simulink model "HvacRecircCtrl"  (R2022b)
settings: Solver=FixedStepDiscrete  StartTime=0.0  StopTime=10.0  FixedStep=0.2  RelTol=1e-3
settings Model: Creator=blue.ge  LastModifiedBy=blue.ge
settings DataIOCC: SaveFormat=StructureWithTime
settings OptimizationCC: DefaultParameterBehavior=Inlined  EnhancedBackFolding=on  PassReuseOutputArgsAs=Individual arguments  ZeroExternalMemoryAtStartup=off  ZeroInternalMemoryAtStartup=off  OptimizeBlockOrder=speed  DifferentSizesBufferReuse=on  OptimizationCustomize=off  EfficientTunableParamExpr=on
settings DebuggingCC: ParameterTunabilityLossMsg=error
settings ModelReferenceCC: ModelReferenceNumInstancesAllowed=Single
settings SFSimCC: MATLABDynamicMemAlloc=off
settings RTWCC: Description=AUTOSAR  SystemTargetFile=autosar.tlc  GenCodeOnly=on  TemplateMakefile=ert_default_tmf
settings CodeAppCC: ForceParamTrailComments=on  IgnoreCustomStorageClasses=off  OperatorAnnotations=on  SimulinkDataObjDesc=on  SFDataObjDesc=on  InsertBlockDesc=on
settings STFCustomTargetCC: TargetLangStandard=C99 (ISO)  MultiwordLength=256  ParMdlRefBuildCompliant=on  CompOptLevelCompliant=on  IncludeMdlTerminateFcn=off  CombineOutputUpdateFcns=on  SuppressErrorStatus=on  ERTCustomFileBanners=on  SupportNonFinite=off  SupportComplex=off  SupportContinuousTime=off  SupportNonInlinedSFcns=off  RemoveResetFunc=on  MATLABClassNameForMDSCustomization=Simulink.SoftwareTarget.ERTCustomization  AutosarCompliant=on  UseToolchainInfoCompliant=on  LookupTableObjectStructAxisOrder=2,1,3,4,...  LUTObjectStructOrderEvenSpacing=Size,Table,Breakpoints  SystemTargetFile=autosar.tlc
settings ERTTargetCC: TargetLangStandard=C99 (ISO)  ConcurrentExecutionCompliant=off  IncludeMdlTerminateFcn=off  SuppressErrorStatus=on  SupportNonFinite=off  SupportComplex=off  ModelStepFunctionPrototypeControlCompliant=off  CPPClassGenCompliant=off  AutosarCompliant=on  LookupTableObjectStructAxisOrder=2,1,3,4,...  LUTObjectStructOrderEvenSpacing=Size,Table,Breakpoints  InlinedParameterPlacement=NonHierarchical  ERTCustomFileTemplate=example_file_process.tlc

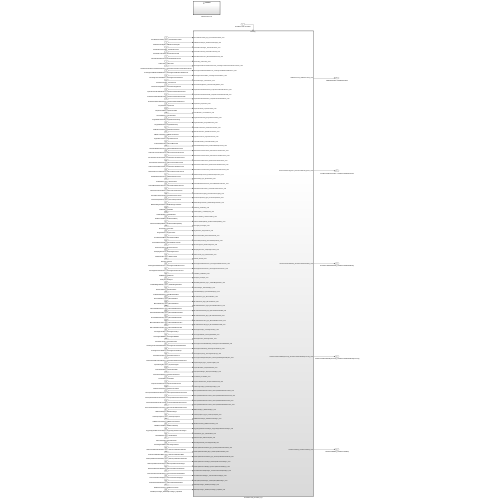
[thumbnail: Simulink's stored preview of the top-level diagram]
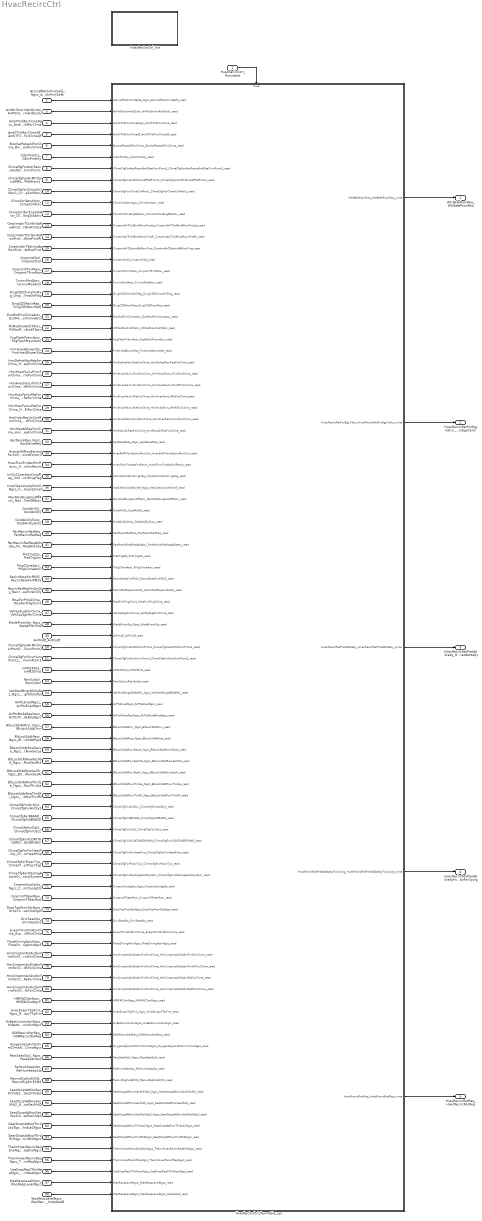
== system HvacRecircCtrl ==
  HvacRecircCtrl_Runnable : Inport  SampleTime=0.2  OutputFunctionCall=on
  ActvnOfWshrFrntSafe_Ngcc_ActvnOfWshrFrntSafe : Inport  Port=2  OutDataTypeStr=Enum: OnOffCrit1  PortDimensions=1
  AirMtrlSnsrInAirQlyIdx_AirMtrlSnsrInAirQlyIdx : Inport  Port=3  OutDataTypeStr=AirMtrlSnsrAirQlyIdx  PortDimensions=1
  AmbTFildForClimaNgcc_AmbTFildForClima : Inport  Port=4  OutDataTypeStr=T30  PortDimensions=1
  AmbTFildForClimaQf_AmbTFildForClimaQf : Inport  Port=5  OutDataTypeStr=Enum: GenQf1  PortDimensions=1
  BiochalPatseldForClima_BiochalPatseldForClima : Inport  Port=6  OutDataTypeStr=Boolean  PortDimensions=1
  CdsnProblty_CdsnProblty : Inport  Port=7  OutDataTypeStr=Fac30  PortDimensions=1
  ClimaCfgForActrRecircAndOsaCmnPrsnt1_ClimaCfgForActrRecircAndOsaCmnPrsnt1 : Inport  Port=8  OutDataTypeStr=Enum: ClimaCfgForActrRecircAndOsaCmnPrsnt1  PortDimensions=1
  ClimaCfgForAirMtrlSnsrAPMAPrsnt1_ClimaCfgForAirMtrlSnsrAPMAPrsnt1 : Inport  Port=9  OutDataTypeStr=Boolean  PortDimensions=1
  ClimaCfgForClimaCtrlMan1_ClimaCfgForClimaCtrlMan1 : Inport  Port=10  OutDataTypeStr=Boolean  PortDimensions=1
  ClimaCtrlActvNgcc_ClimaCtrlActv : Inport  Port=11  OutDataTypeStr=Boolean  PortDimensions=1
  ClimaCtrlForEngStbActv_ClimaCtrlForEngStbActv : Inport  Port=12  OutDataTypeStr=Boolean  PortDimensions=1
  CmptmtAirTCtrlErrAtRowFirstLe_CmptmtAirTCtrlErrAtRowFirstLe : Inport  Port=13  OutDataTypeStr=T30  PortDimensions=1
  CmptmtAirTCtrlErrAtRowFirstRi_CmptmtAirTCtrlErrAtRowFirstRi : Inport  Port=14  OutDataTypeStr=T30  PortDimensions=1
  CmptmtAirTEstimdAtRowFirst_CmptmtAirTEstimdAtRowFirst : Inport  Port=15  OutDataTypeStr=T30  PortDimensions=1
  CmptmtClsd_CmptmtClsd : Inport  Port=16  OutDataTypeStr=Boolean  PortDimensions=1
  CmptmtTFrntRaw_CmptmtTFrntRaw : Inport  Port=17  OutDataTypeStr=Bus: CmptmtTFrnt  PortDimensions=1  BusOutputAsStruct=on
  CnvincModActv_CnvincModActv : Inport  Port=18  OutDataTypeStr=Boolean  PortDimensions=1
  DrvgCDSClimaOnFlag_DrvgCDSClimaOnFlag : Inport  Port=19  OutDataTypeStr=Boolean  PortDimensions=1
  DrvgCDSRecirReq_DrvgCDSRecirReq : Inport  Port=20  OutDataTypeStr=Perc30  PortDimensions=1
  EcoModForClimaActv_EcoModForClimaActv : Inport  Port=21  OutDataTypeStr=Enum: OnOff1  PortDimensions=1
  FltModForAmbTActv_FltModForAmbTActv : Inport  Port=22  OutDataTypeStr=Boolean  PortDimensions=1
  FogFlashPrevnActv_FogFlashPrevnActv : Inport  Port=23  OutDataTypeStr=Boolean  PortDimensions=1
  FrntHvacBlowerSts_FrntHvacBlowerSts : Inport  Port=24  OutDataTypeStr=Enum: HvacFanSts  PortDimensions=1
  HmiDefrstMaxReqForClima_HmiDefrstMaxReqForClima : Inport  Port=25  OutDataTypeStr=Enum: ActrReq  PortDimensions=1
  HmiHvacFanLvlFrntForClima_HmiHvacFanLvlFrntForClima : Inport  Port=26  OutDataTypeStr=Enum: HmiHvacFanLvl  PortDimensions=1
  HmiHvacFanLvlFrntForClima_HmiHvacFanLvlFrntRiForClima : Inport  Port=27  OutDataTypeStr=Enum: HmiHvacFanLvl  PortDimensions=1
  HmiHvacFanLvlReForClima_HmiHvacFanLvlReForClima : Inport  Port=28  OutDataTypeStr=Enum: HmiHvacFanLvl  PortDimensions=1
  HmiHvacFanLvlReForClima_HmiHvacFanLvlReRiForClima : Inport  Port=29  OutDataTypeStr=Enum: HmiHvacFanLvl  PortDimensions=1
  HmiHvacRecircCmdForClima_HmiHvacRecircCmdForClima : Inport  Port=30  OutDataTypeStr=Enum: HmiHvacRecircCmd  PortDimensions=1
  HmiMaxACReqForClima_HmiMaxACReqForClima : Inport  Port=31  OutDataTypeStr=Enum: OnOff1  PortDimensions=1
  HpcDeiceReq_Ngcc_HpcDeiceReq : Inport  Port=32  OutDataTypeStr=Enum: DeiceReq  PortDimensions=1
  HvacAirMFlowEstimdForCtrlr_HvacAirMFlowEstimdForCtrlr : Inport  Port=33  OutDataTypeStr=AirFlow30  PortDimensions=1
  HvacFlowFrntActForRecirc_HvacFlowFrntActForRecirc : Inport  Port=34  OutDataTypeStr=AirFlow30  PortDimensions=1
  IntCo2SnsrActvDrvgFlag_IntCo2SnsrActvDrvgFlag : Inport  Port=35  OutDataTypeStr=Boolean  PortDimensions=1
  InteCleanUnpleSmell_Ngcc_InteCleanUnpleSmell : Inport  Port=36  OutDataTypeStr=Enum: OnOff1  PortDimensions=1
  ManModDurgFanOffActv_ManModDurgFanOffActv : Inport  Port=37  OutDataTypeStr=Boolean  PortDimensions=1
  OutdAirQly_OutdAirQly : Inport  Port=38  OutDataTypeStr=Bus: OutdAirQly  PortDimensions=1  BusOutputAsStruct=on
  OutdAirQlyDuty_OutdAirQlyDuty : Inport  Port=39  OutDataTypeStr=Bus: OutdAirQlyDutyWithQly  PortDimensions=1  BusOutputAsStruct=on
  PartRecircRatReq_PartRecircRatReq : Inport  Port=40  OutDataTypeStr=Perc30  PortDimensions=1
  PartRecircRatReqAtStaby_PartRecircRatReqAtStaby : Inport  Port=41  OutDataTypeStr=Perc30  PortDimensions=1
  PreClngSts_PreClngSts : Inport  Port=42  OutDataTypeStr=Enum: PreClngSts  PortDimensions=1
  PrkgClimaActv_PrkgClimaActv : Inport  Port=43  OutDataTypeStr=Enum: PrkgClimaActv  PortDimensions=1
  RecircRateForPM25_RecircRateForPM25 : Inport  Port=44  OutDataTypeStr=Perc30  PortDimensions=1
  RecircRatReqForAirQly_RecircRatReqForAirQly : Inport  Port=45  OutDataTypeStr=Perc30  PortDimensions=1
  RtLeForPrkgClima_RtLeForPrkgClima : Inport  Port=46  OutDataTypeStr=Ti33  PortDimensions=1
  VehSpdLgtForClima_VehSpdLgtForClima : Inport  Port=47  OutDataTypeStr=Bus: VehSpdLgtForClima  PortDimensions=1  BusOutputAsStruct=on
  WadeProtnSts_Ngcc_WadeProtnSts : Inport  Port=48  OutDataTypeStr=Enum: OnOff1  PortDimensions=1
  AirPmQf_AirPmQf : Inport  Port=49  OutDataTypeStr=Enum: AirPmQf  PortDimensions=1
  ClimaCfgForAirMtrlSnsrPrsnt1_ClimaCfgForAirMtrlSnsrPrsnt1 : Inport  Port=50  OutDataTypeStr=Enum: CarCfgForAirMtrlSnsrGly  PortDimensions=1
  ClimaCfgForSnsrHumPrsnt1_ClimaCfgForSnsrHumPrsnt1 : Inport  Port=51  OutDataTypeStr=Boolean  PortDimensions=1
  InPM25Fild_InPM25Fild : Inport  Port=52  OutDataTypeStr=UInt10  PortDimensions=1
  RainSybol_RainSybol : Inport  Port=53  OutDataTypeStr=Boolean  PortDimensions=1
  VehModMngtGlbSafe1_Ngcc_VehModMngtGlbSafe1 : Inport  Port=54  OutDataTypeStr=Bus: VehModMngtGlbSafe1  PortDimensions=1  BusOutputAsStruct=on
  AirPtcEnadNgcc_AirPtcEnadNgcc : Inport  Port=55  OutDataTypeStr=Boolean  PortDimensions=1
  AirPtcModeReqNgcc_AirPtcModeReqNgcc : Inport  Port=56  OutDataTypeStr=Enum: AirPtcModeReq  PortDimensions=1
  BltLockStAtDrvr_Ngcc_BltLockStAtDrvr : Inport  Port=57  OutDataTypeStr=Bus: BltLockStFrnt  PortDimensions=1  BusOutputAsStruct=on
  BltLockStAtPass_Ngcc_BltLockStAtPass : Inport  Port=58  OutDataTypeStr=Bus: BltLockStFrnt  PortDimensions=1  BusOutputAsStruct=on
  BltLockStAtRowSecLe_Ngcc_BltLockStAtRowSecLe : Inport  Port=59  OutDataTypeStr=Bus: BltLockStRe  PortDimensions=1  BusOutputAsStruct=on
  BltLockStAtRowSecMid_Ngcc_BltLockStAtRowSecMid : Inport  Port=60  OutDataTypeStr=Bus: BltLockStRe  PortDimensions=1  BusOutputAsStruct=on
  BltLockStAtRowSecRi_Ngcc_BltLockStAtRowSecRi : Inport  Port=61  OutDataTypeStr=Bus: BltLockStRe  PortDimensions=1  BusOutputAsStruct=on
  BltLockStAtRowThrdLe_Ngcc_BltLockStAtRowThrdLe : Inport  Port=62  OutDataTypeStr=Bus: BltLockStRe  PortDimensions=1  BusOutputAsStruct=on
  BltLockStAtRowThrdRi_Ngcc_BltLockStAtRowThrdRi : Inport  Port=63  OutDataTypeStr=Bus: BltLockStRe  PortDimensions=1  BusOutputAsStruct=on
  ClimaCfgForAirQly1_ClimaCfgForAirQly1 : Inport  Port=64  OutDataTypeStr=Boolean  PortDimensions=1
  ClimaCfgForBRAND_ClimaCfgForBRAND : Inport  Port=65  OutDataTypeStr=Enum: ClimaCfgForBRAND  PortDimensions=1
  ClimaCfgForCds1_ClimaCfgForCds1 : Inport  Port=66  OutDataTypeStr=Boolean  PortDimensions=1
  ClimaCfgForFLOATINGDRVING_ClimaCfgForFLOATINGDRVING : Inport  Port=67  OutDataTypeStr=Enum: FLOATINGDRVING  PortDimensions=1
  ClimaCfgForForHeatPmp_ClimaCfgForForHeatPmp : Inport  Port=68  OutDataTypeStr=Enum: CarCfgForHeatPmpTyp  PortDimensions=1
  ClimaCfgForPrpsnTyp_ClimaCfgForPrpsnTyp : Inport  Port=69  OutDataTypeStr=Enum: CarCfgForPrpsnTypGly  PortDimensions=1
  ClimaCfgForWadingAssistSystem_ClimaCfgForWadingAssistSystem : Inport  Port=70  OutDataTypeStr=Enum: WadingAssistSystem  PortDimensions=1
  CmptmtCoolgSts_Ngcc_CmptmtCoolgSts : Inport  Port=71  OutDataTypeStr=Enum: CmptmtCoolgSts  PortDimensions=1
  CmptmtTRearRaw_CmptmtTRearRaw : Inport  Port=72  OutDataTypeStr=Bus: CmptmtTFrnt  PortDimensions=1  BusOutputAsStruct=on
  DropTopPosnStsNgcc_DropTopPosnStsNgcc : Inport  Port=73  OutDataTypeStr=Enum: PosnSts  PortDimensions=1
  DrvrSeatSts_DrvrSeatSts : Inport  Port=74  OutDataTypeStr=Enum: OccptPresSt1  PortDimensions=1
  EvaprTFrntFildForClima_EvaprTFrntFildForClima : Inport  Port=75  OutDataTypeStr=T30  PortDimensions=1
  FloatDrvingActvNgcc_FloatDrvingActvNgcc : Inport  Port=76  OutDataTypeStr=Bus: FloatDrvingActv  PortDimensions=1  BusOutputAsStruct=on
  HmiCmptmtAirDistbnFrntForClima_HmiCmptmtAirDistbnFrntForClima : Inport  Port=77  OutDataTypeStr=Enum: HmiCmptmtAirDistbnFrnt  PortDimensions=1
  HmiCmptmtAirDistbnFrntForClima_HmiCmptmtAirDistbnFrntRiForClima : Inport  Port=78  OutDataTypeStr=Enum: HmiCmptmtAirDistbnFrnt  PortDimensions=1
  HmiCmptmtAirDistbnFrntForClima_HmiCmptmtAirDistbnReForClima : Inport  Port=79  OutDataTypeStr=Enum: HmiCmptmtAirDistbnFrnt  PortDimensions=1
  HmiCmptmtAirDistbnFrntForClima_HmiCmptmtAirDistbnReRiForClima : Inport  Port=80  OutDataTypeStr=Enum: HmiCmptmtAirDistbnFrnt  PortDimensions=1
  HMIFACSwtNgcc_HMIFACSwtNgcc : Inport  Port=81  OutDataTypeStr=Enum: OnOff1  PortDimensions=1
  HvacEvaprTSpFrnt_Ngcc_HvacEvaprTSpFrnt : Inport  Port=82  OutDataTypeStr=HvacEvaprTSp  PortDimensions=1
  HvBattLimnIndcnNgcc_HvBattLimnIndcnNgcc : Inport  Port=83  OutDataTypeStr=UInt16  PortDimensions=1
  OSMRecircRatReq_OSMRecircRatReq : Inport  Port=84  OutMin=0  OutMax=2047  OutDataTypeStr=Pwr30  PortDimensions=1
  OxygenSplyAvlStsFrmClimaNgcc_OxygenSplyAvlStsFrmClimaNgcc : Inport  Port=85  OutDataTypeStr=Enum: OxygenSplyAvlSts  PortDimensions=1
  PassSeatSts2_Ngcc_PassSeatSts2 : Inport  Port=86  OutDataTypeStr=Enum: OccptPresSt1  PortDimensions=1
  RelHumKeepSts_RelHumKeepSts : Inport  Port=87  OutDataTypeStr=Enum: RelHumKeepSts  PortDimensions=1
  ResrvdSigForECM4_ResrvdSigForECM4 : Inport  Port=88  OutDataTypeStr=BattSnsrChksTbl  PortDimensions=1
  SeatOccptAtRowSecDrSide2_Ngcc_SeatOccptAtRowSecDrSide2 : Inport  Port=89  OutDataTypeStr=Enum: OccptPresSt1  PortDimensions=1
  SeatOccptAtRowSecMid2_Ngcc_SeatOccptAtRowSecMid2 : Inport  Port=90  OutDataTypeStr=Enum: OccptPresSt1  PortDimensions=1
  SeatOccptAtRowSecPasSide2_Ngcc_SeatOccptAtRowSecPasSide2 : Inport  Port=91  OutDataTypeStr=Enum: OccptPresSt1  PortDimensions=1
  SeatOccptAtRowThrdLe2Ngcc_SeatOccptAtRowThrdLe2Ngcc : Inport  Port=92  OutDataTypeStr=Enum: OccptPresSt1  PortDimensions=1
  SeatOccptAtRowThrdRi2Ngcc_SeatOccptAtRowThrdRi2Ngcc : Inport  Port=93  OutDataTypeStr=Enum: OccptPresSt1  PortDimensions=1
  ThermHvacRecircReqEnaNgcc_ThermHvacRecircReqEnaNgcc : Inport  Port=94  OutDataTypeStr=Boolean  PortDimensions=1
  ThermHvacRecircReqNgcc_ThermHvacRecircReqNgcc : Inport  Port=95  OutMin=0  OutMax=100  OutDataTypeStr=Perc6  PortDimensions=1
  UseEvapReqTMinNewNgcc_UseEvapReqTMinNewNgcc : Inport  Port=96  OutDataTypeStr=Enum: OnOff1  PortDimensions=1
  WasRespLevelNgcc_WasRespLevelNgcc : Inport  Port=97  OutDataTypeStr=Enum: WasRespLevel  PortDimensions=1
  WasRespLevelNgcc_WasRespLevelNgcc_IsUpdated : Inport  Port=98  OutDataTypeStr=boolean  PortDimensions=1
  HvacRecircCtrl_Init : Subsystem
  HvacRecircCtrl_Runnable_sys : Subsystem
  WtrBafePsonReq_WtrBafePsonReq : Outport  OutDataTypeStr=UInt16  PortDimensions=1
  HvacRecircRatForEgyCalcn_HvacRecircRatForEgyCalcn : Outport  Port=2  OutDataTypeStr=Perc30  PortDimensions=1
  HvacRecircRatPredAtStaby_HvacRecircRatPredAtStaby : Outport  Port=3  OutDataTypeStr=Perc30  PortDimensions=1
  HvacRecircRatPredAtStabyForCoolg_HvacRecircRatPredAtStabyForCoolg : Outport  Port=4  OutDataTypeStr=Perc30  PortDimensions=1
  HvacRecircRatReq_HvacRecircRatReq : Outport  Port=5  OutDataTypeStr=Perc30  PortDimensions=1
  wires:
  ActvnOfWshrFrntSafe_Ngcc_ActvnOfWshrFrntSafe/1 -> HvacRecircCtrl_Runnable_sys/1(ActvnOfWshrFrntSafe_Ngcc_ActvnOfWshrFrntSafe_read)
  AirMtrlSnsrInAirQlyIdx_AirMtrlSnsrInAirQlyIdx/1 -> HvacRecircCtrl_Runnable_sys/2(AirMtrlSnsrInAirQlyIdx_AirMtrlSnsrInAirQlyIdx_read)
  AmbTFildForClimaNgcc_AmbTFildForClima/1 -> HvacRecircCtrl_Runnable_sys/3(AmbTFildForClimaNgcc_AmbTFildForClima_read)
  AmbTFildForClimaQf_AmbTFildForClimaQf/1 -> HvacRecircCtrl_Runnable_sys/4(AmbTFildForClimaQf_AmbTFildForClimaQf_read)
  BiochalPatseldForClima_BiochalPatseldForClima/1 -> HvacRecircCtrl_Runnable_sys/5(BiochalPatseldForClima_BiochalPatseldForClima_read)
  CdsnProblty_CdsnProblty/1 -> HvacRecircCtrl_Runnable_sys/6(CdsnProblty_CdsnProblty_read)
  ClimaCfgForActrRecircAndOsaCmnPrsnt1_ClimaCfgForActrRecircAndOsaCmnPrsnt1/1 -> HvacRecircCtrl_Runnable_sys/7(ClimaCfgForActrRecircAndOsaCmnPrsnt1_ClimaCfgForActrRecircAndOsaCmnPrsnt1_read)
  ClimaCfgForAirMtrlSnsrAPMAPrsnt1_ClimaCfgForAirMtrlSnsrAPMAPrsnt1/1 -> HvacRecircCtrl_Runnable_sys/8(ClimaCfgForAirMtrlSnsrAPMAPrsnt1_ClimaCfgForAirMtrlSnsrAPMAPrsnt1_read)
  ClimaCfgForClimaCtrlMan1_ClimaCfgForClimaCtrlMan1/1 -> HvacRecircCtrl_Runnable_sys/9(ClimaCfgForClimaCtrlMan1_ClimaCfgForClimaCtrlMan1_read)
  ClimaCtrlActvNgcc_ClimaCtrlActv/1 -> HvacRecircCtrl_Runnable_sys/10(ClimaCtrlActvNgcc_ClimaCtrlActv_read)
  ClimaCtrlForEngStbActv_ClimaCtrlForEngStbActv/1 -> HvacRecircCtrl_Runnable_sys/11(ClimaCtrlForEngStbActv_ClimaCtrlForEngStbActv_read)
  CmptmtAirTCtrlErrAtRowFirstLe_CmptmtAirTCtrlErrAtRowFirstLe/1 -> HvacRecircCtrl_Runnable_sys/12(CmptmtAirTCtrlErrAtRowFirstLe_CmptmtAirTCtrlErrAtRowFirstLe_read)
  CmptmtAirTCtrlErrAtRowFirstRi_CmptmtAirTCtrlErrAtRowFirstRi/1 -> HvacRecircCtrl_Runnable_sys/13(CmptmtAirTCtrlErrAtRowFirstRi_CmptmtAirTCtrlErrAtRowFirstRi_read)
  CmptmtAirTEstimdAtRowFirst_CmptmtAirTEstimdAtRowFirst/1 -> HvacRecircCtrl_Runnable_sys/14(CmptmtAirTEstimdAtRowFirst_CmptmtAirTEstimdAtRowFirst_read)
  CmptmtClsd_CmptmtClsd/1 -> HvacRecircCtrl_Runnable_sys/15(CmptmtClsd_CmptmtClsd_read)
  CmptmtTFrntRaw_CmptmtTFrntRaw/1 -> HvacRecircCtrl_Runnable_sys/16(CmptmtTFrntRaw_CmptmtTFrntRaw_read)
  CnvincModActv_CnvincModActv/1 -> HvacRecircCtrl_Runnable_sys/17(CnvincModActv_CnvincModActv_read)
  DrvgCDSClimaOnFlag_DrvgCDSClimaOnFlag/1 -> HvacRecircCtrl_Runnable_sys/18(DrvgCDSClimaOnFlag_DrvgCDSClimaOnFlag_read)
  DrvgCDSRecirReq_DrvgCDSRecirReq/1 -> HvacRecircCtrl_Runnable_sys/19(DrvgCDSRecirReq_DrvgCDSRecirReq_read)
  EcoModForClimaActv_EcoModForClimaActv/1 -> HvacRecircCtrl_Runnable_sys/20(EcoModForClimaActv_EcoModForClimaActv_read)
  FltModForAmbTActv_FltModForAmbTActv/1 -> HvacRecircCtrl_Runnable_sys/21(FltModForAmbTActv_FltModForAmbTActv_read)
  FogFlashPrevnActv_FogFlashPrevnActv/1 -> HvacRecircCtrl_Runnable_sys/22(FogFlashPrevnActv_FogFlashPrevnActv_read)
  FrntHvacBlowerSts_FrntHvacBlowerSts/1 -> HvacRecircCtrl_Runnable_sys/23(FrntHvacBlowerSts_FrntHvacBlowerSts_read)
  HmiDefrstMaxReqForClima_HmiDefrstMaxReqForClima/1 -> HvacRecircCtrl_Runnable_sys/24(HmiDefrstMaxReqForClima_HmiDefrstMaxReqForClima_read)
  HmiHvacFanLvlFrntForClima_HmiHvacFanLvlFrntForClima/1 -> HvacRecircCtrl_Runnable_sys/25(HmiHvacFanLvlFrntForClima_HmiHvacFanLvlFrntForClima_read)
  HmiHvacFanLvlFrntForClima_HmiHvacFanLvlFrntRiForClima/1 -> HvacRecircCtrl_Runnable_sys/26(HmiHvacFanLvlFrntForClima_HmiHvacFanLvlFrntRiForClima_read)
  HmiHvacFanLvlReForClima_HmiHvacFanLvlReForClima/1 -> HvacRecircCtrl_Runnable_sys/27(HmiHvacFanLvlReForClima_HmiHvacFanLvlReForClima_read)
  HmiHvacFanLvlReForClima_HmiHvacFanLvlReRiForClima/1 -> HvacRecircCtrl_Runnable_sys/28(HmiHvacFanLvlReForClima_HmiHvacFanLvlReRiForClima_read)
  HmiHvacRecircCmdForClima_HmiHvacRecircCmdForClima/1 -> HvacRecircCtrl_Runnable_sys/29(HmiHvacRecircCmdForClima_HmiHvacRecircCmdForClima_read)
  HmiMaxACReqForClima_HmiMaxACReqForClima/1 -> HvacRecircCtrl_Runnable_sys/30(HmiMaxACReqForClima_HmiMaxACReqForClima_read)
  HpcDeiceReq_Ngcc_HpcDeiceReq/1 -> HvacRecircCtrl_Runnable_sys/31(HpcDeiceReq_Ngcc_HpcDeiceReq_read)
  HvacAirMFlowEstimdForCtrlr_HvacAirMFlowEstimdForCtrlr/1 -> HvacRecircCtrl_Runnable_sys/32(HvacAirMFlowEstimdForCtrlr_HvacAirMFlowEstimdForCtrlr_read)
  HvacFlowFrntActForRecirc_HvacFlowFrntActForRecirc/1 -> HvacRecircCtrl_Runnable_sys/33(HvacFlowFrntActForRecirc_HvacFlowFrntActForRecirc_read)
  IntCo2SnsrActvDrvgFlag_IntCo2SnsrActvDrvgFlag/1 -> HvacRecircCtrl_Runnable_sys/34(IntCo2SnsrActvDrvgFlag_IntCo2SnsrActvDrvgFlag_read)
  InteCleanUnpleSmell_Ngcc_InteCleanUnpleSmell/1 -> HvacRecircCtrl_Runnable_sys/35(InteCleanUnpleSmell_Ngcc_InteCleanUnpleSmell_read)
  ManModDurgFanOffActv_ManModDurgFanOffActv/1 -> HvacRecircCtrl_Runnable_sys/36(ManModDurgFanOffActv_ManModDurgFanOffActv_read)
  OutdAirQly_OutdAirQly/1 -> HvacRecircCtrl_Runnable_sys/37(OutdAirQly_OutdAirQly_read)
  OutdAirQlyDuty_OutdAirQlyDuty/1 -> HvacRecircCtrl_Runnable_sys/38(OutdAirQlyDuty_OutdAirQlyDuty_read)
  PartRecircRatReq_PartRecircRatReq/1 -> HvacRecircCtrl_Runnable_sys/39(PartRecircRatReq_PartRecircRatReq_read)
  PartRecircRatReqAtStaby_PartRecircRatReqAtStaby/1 -> HvacRecircCtrl_Runnable_sys/40(PartRecircRatReqAtStaby_PartRecircRatReqAtStaby_read)
  PreClngSts_PreClngSts/1 -> HvacRecircCtrl_Runnable_sys/41(PreClngSts_PreClngSts_read)
  PrkgClimaActv_PrkgClimaActv/1 -> HvacRecircCtrl_Runnable_sys/42(PrkgClimaActv_PrkgClimaActv_read)
  RecircRateForPM25_RecircRateForPM25/1 -> HvacRecircCtrl_Runnable_sys/43(RecircRateForPM25_RecircRateForPM25_read)
  RecircRatReqForAirQly_RecircRatReqForAirQly/1 -> HvacRecircCtrl_Runnable_sys/44(RecircRatReqForAirQly_RecircRatReqForAirQly_read)
  RtLeForPrkgClima_RtLeForPrkgClima/1 -> HvacRecircCtrl_Runnable_sys/45(RtLeForPrkgClima_RtLeForPrkgClima_read)
  VehSpdLgtForClima_VehSpdLgtForClima/1 -> HvacRecircCtrl_Runnable_sys/46(VehSpdLgtForClima_VehSpdLgtForClima_read)
  WadeProtnSts_Ngcc_WadeProtnSts/1 -> HvacRecircCtrl_Runnable_sys/47(WadeProtnSts_Ngcc_WadeProtnSts_read)
  AirPmQf_AirPmQf/1 -> HvacRecircCtrl_Runnable_sys/48(AirPmQf_AirPmQf_read)
  ClimaCfgForAirMtrlSnsrPrsnt1_ClimaCfgForAirMtrlSnsrPrsnt1/1 -> HvacRecircCtrl_Runnable_sys/49(ClimaCfgForAirMtrlSnsrPrsnt1_ClimaCfgForAirMtrlSnsrPrsnt1_read)
  ClimaCfgForSnsrHumPrsnt1_ClimaCfgForSnsrHumPrsnt1/1 -> HvacRecircCtrl_Runnable_sys/50(ClimaCfgForSnsrHumPrsnt1_ClimaCfgForSnsrHumPrsnt1_read)
  InPM25Fild_InPM25Fild/1 -> HvacRecircCtrl_Runnable_sys/51(InPM25Fild_InPM25Fild_read)
  RainSybol_RainSybol/1 -> HvacRecircCtrl_Runnable_sys/52(RainSybol_RainSybol_read)
  VehModMngtGlbSafe1_Ngcc_VehModMngtGlbSafe1/1 -> HvacRecircCtrl_Runnable_sys/53(VehModMngtGlbSafe1_Ngcc_VehModMngtGlbSafe1_read)
  AirPtcEnadNgcc_AirPtcEnadNgcc/1 -> HvacRecircCtrl_Runnable_sys/54(AirPtcEnadNgcc_AirPtcEnadNgcc_read)
  AirPtcModeReqNgcc_AirPtcModeReqNgcc/1 -> HvacRecircCtrl_Runnable_sys/55(AirPtcModeReqNgcc_AirPtcModeReqNgcc_read)
  BltLockStAtDrvr_Ngcc_BltLockStAtDrvr/1 -> HvacRecircCtrl_Runnable_sys/56(BltLockStAtDrvr_Ngcc_BltLockStAtDrvr_read)
  BltLockStAtPass_Ngcc_BltLockStAtPass/1 -> HvacRecircCtrl_Runnable_sys/57(BltLockStAtPass_Ngcc_BltLockStAtPass_read)
  BltLockStAtRowSecLe_Ngcc_BltLockStAtRowSecLe/1 -> HvacRecircCtrl_Runnable_sys/58(BltLockStAtRowSecLe_Ngcc_BltLockStAtRowSecLe_read)
  BltLockStAtRowSecMid_Ngcc_BltLockStAtRowSecMid/1 -> HvacRecircCtrl_Runnable_sys/59(BltLockStAtRowSecMid_Ngcc_BltLockStAtRowSecMid_read)
  BltLockStAtRowSecRi_Ngcc_BltLockStAtRowSecRi/1 -> HvacRecircCtrl_Runnable_sys/60(BltLockStAtRowSecRi_Ngcc_BltLockStAtRowSecRi_read)
  BltLockStAtRowThrdLe_Ngcc_BltLockStAtRowThrdLe/1 -> HvacRecircCtrl_Runnable_sys/61(BltLockStAtRowThrdLe_Ngcc_BltLockStAtRowThrdLe_read)
  BltLockStAtRowThrdRi_Ngcc_BltLockStAtRowThrdRi/1 -> HvacRecircCtrl_Runnable_sys/62(BltLockStAtRowThrdRi_Ngcc_BltLockStAtRowThrdRi_read)
  ClimaCfgForAirQly1_ClimaCfgForAirQly1/1 -> HvacRecircCtrl_Runnable_sys/63(ClimaCfgForAirQly1_ClimaCfgForAirQly1_read)
  ClimaCfgForBRAND_ClimaCfgForBRAND/1 -> HvacRecircCtrl_Runnable_sys/64(ClimaCfgForBRAND_ClimaCfgForBRAND_read)
  ClimaCfgForCds1_ClimaCfgForCds1/1 -> HvacRecircCtrl_Runnable_sys/65(ClimaCfgForCds1_ClimaCfgForCds1_read)
  ClimaCfgForFLOATINGDRVING_ClimaCfgForFLOATINGDRVING/1 -> HvacRecircCtrl_Runnable_sys/66(ClimaCfgForFLOATINGDRVING_ClimaCfgForFLOATINGDRVING_read)
  ClimaCfgForForHeatPmp_ClimaCfgForForHeatPmp/1 -> HvacRecircCtrl_Runnable_sys/67(ClimaCfgForForHeatPmp_ClimaCfgForForHeatPmp_read)
  ClimaCfgForPrpsnTyp_ClimaCfgForPrpsnTyp/1 -> HvacRecircCtrl_Runnable_sys/68(ClimaCfgForPrpsnTyp_ClimaCfgForPrpsnTyp_read)
  ClimaCfgForWadingAssistSystem_ClimaCfgForWadingAssistSystem/1 -> HvacRecircCtrl_Runnable_sys/69(ClimaCfgForWadingAssistSystem_ClimaCfgForWadingAssistSystem_read)
  CmptmtCoolgSts_Ngcc_CmptmtCoolgSts/1 -> HvacRecircCtrl_Runnable_sys/70(CmptmtCoolgSts_Ngcc_CmptmtCoolgSts_read)
  CmptmtTRearRaw_CmptmtTRearRaw/1 -> HvacRecircCtrl_Runnable_sys/71(CmptmtTRearRaw_CmptmtTRearRaw_read)
  DropTopPosnStsNgcc_DropTopPosnStsNgcc/1 -> HvacRecircCtrl_Runnable_sys/72(DropTopPosnStsNgcc_DropTopPosnStsNgcc_read)
  DrvrSeatSts_DrvrSeatSts/1 -> HvacRecircCtrl_Runnable_sys/73(DrvrSeatSts_DrvrSeatSts_read)
  EvaprTFrntFildForClima_EvaprTFrntFildForClima/1 -> HvacRecircCtrl_Runnable_sys/74(EvaprTFrntFildForClima_EvaprTFrntFildForClima_read)
  FloatDrvingActvNgcc_FloatDrvingActvNgcc/1 -> HvacRecircCtrl_Runnable_sys/75(FloatDrvingActvNgcc_FloatDrvingActvNgcc_read)
  HmiCmptmtAirDistbnFrntForClima_HmiCmptmtAirDistbnFrntForClima/1 -> HvacRecircCtrl_Runnable_sys/76(HmiCmptmtAirDistbnFrntForClima_HmiCmptmtAirDistbnFrntForClima_read)
  HmiCmptmtAirDistbnFrntForClima_HmiCmptmtAirDistbnFrntRiForClima/1 -> HvacRecircCtrl_Runnable_sys/77(HmiCmptmtAirDistbnFrntForClima_HmiCmptmtAirDistbnFrntRiForClima_read)
  HmiCmptmtAirDistbnFrntForClima_HmiCmptmtAirDistbnReForClima/1 -> HvacRecircCtrl_Runnable_sys/78(HmiCmptmtAirDistbnFrntForClima_HmiCmptmtAirDistbnReForClima_read)
  HmiCmptmtAirDistbnFrntForClima_HmiCmptmtAirDistbnReRiForClima/1 -> HvacRecircCtrl_Runnable_sys/79(HmiCmptmtAirDistbnFrntForClima_HmiCmptmtAirDistbnReRiForClima_read)
  HMIFACSwtNgcc_HMIFACSwtNgcc/1 -> HvacRecircCtrl_Runnable_sys/80(HMIFACSwtNgcc_HMIFACSwtNgcc_read)
  HvacEvaprTSpFrnt_Ngcc_HvacEvaprTSpFrnt/1 -> HvacRecircCtrl_Runnable_sys/81(HvacEvaprTSpFrnt_Ngcc_HvacEvaprTSpFrnt_read)
  HvBattLimnIndcnNgcc_HvBattLimnIndcnNgcc/1 -> HvacRecircCtrl_Runnable_sys/82(HvBattLimnIndcnNgcc_HvBattLimnIndcnNgcc_read)
  OSMRecircRatReq_OSMRecircRatReq/1 -> HvacRecircCtrl_Runnable_sys/83(OSMRecircRatReq_OSMRecircRatReq_read)
  OxygenSplyAvlStsFrmClimaNgcc_OxygenSplyAvlStsFrmClimaNgcc/1 -> HvacRecircCtrl_Runnable_sys/84(OxygenSplyAvlStsFrmClimaNgcc_OxygenSplyAvlStsFrmClimaNgcc_read)
  PassSeatSts2_Ngcc_PassSeatSts2/1 -> HvacRecircCtrl_Runnable_sys/85(PassSeatSts2_Ngcc_PassSeatSts2_read)
  RelHumKeepSts_RelHumKeepSts/1 -> HvacRecircCtrl_Runnable_sys/86(RelHumKeepSts_RelHumKeepSts_read)
  ResrvdSigForECM4_ResrvdSigForECM4/1 -> HvacRecircCtrl_Runnable_sys/87(ResrvdSigForECM4_ResrvdSigForECM4_read)
  SeatOccptAtRowSecDrSide2_Ngcc_SeatOccptAtRowSecDrSide2/1 -> HvacRecircCtrl_Runnable_sys/88(SeatOccptAtRowSecDrSide2_Ngcc_SeatOccptAtRowSecDrSide2_read)
  SeatOccptAtRowSecMid2_Ngcc_SeatOccptAtRowSecMid2/1 -> HvacRecircCtrl_Runnable_sys/89(SeatOccptAtRowSecMid2_Ngcc_SeatOccptAtRowSecMid2_read)
  SeatOccptAtRowSecPasSide2_Ngcc_SeatOccptAtRowSecPasSide2/1 -> HvacRecircCtrl_Runnable_sys/90(SeatOccptAtRowSecPasSide2_Ngcc_SeatOccptAtRowSecPasSide2_read)
  SeatOccptAtRowThrdLe2Ngcc_SeatOccptAtRowThrdLe2Ngcc/1 -> HvacRecircCtrl_Runnable_sys/91(SeatOccptAtRowThrdLe2Ngcc_SeatOccptAtRowThrdLe2Ngcc_read)
  SeatOccptAtRowThrdRi2Ngcc_SeatOccptAtRowThrdRi2Ngcc/1 -> HvacRecircCtrl_Runnable_sys/92(SeatOccptAtRowThrdRi2Ngcc_SeatOccptAtRowThrdRi2Ngcc_read)
  ThermHvacRecircReqEnaNgcc_ThermHvacRecircReqEnaNgcc/1 -> HvacRecircCtrl_Runnable_sys/93(ThermHvacRecircReqEnaNgcc_ThermHvacRecircReqEnaNgcc_read)
  ThermHvacRecircReqNgcc_ThermHvacRecircReqNgcc/1 -> HvacRecircCtrl_Runnable_sys/94(ThermHvacRecircReqNgcc_ThermHvacRecircReqNgcc_read)
  UseEvapReqTMinNewNgcc_UseEvapReqTMinNewNgcc/1 -> HvacRecircCtrl_Runnable_sys/95(UseEvapReqTMinNewNgcc_UseEvapReqTMinNewNgcc_read)
  WasRespLevelNgcc_WasRespLevelNgcc/1 -> HvacRecircCtrl_Runnable_sys/96(WasRespLevelNgcc_WasRespLevelNgcc_read)
  WasRespLevelNgcc_WasRespLevelNgcc_IsUpdated/1 -> HvacRecircCtrl_Runnable_sys/97(WasRespLevelNgcc_WasRespLevelNgcc_IsUpdated_read)
  HvacRecircCtrl_Runnable_sys/1(WtrBafePsonReq_WtrBafePsonReq_write) -> WtrBafePsonReq_WtrBafePsonReq/1
  HvacRecircCtrl_Runnable_sys/2(HvacRecircRatForEgyCalcn_HvacRecircRatForEgyCalcn_write) -> HvacRecircRatForEgyCalcn_HvacRecircRatForEgyCalcn/1
  HvacRecircCtrl_Runnable_sys/3(HvacRecircRatPredAtStaby_HvacRecircRatPredAtStaby_write) -> HvacRecircRatPredAtStaby_HvacRecircRatPredAtStaby/1
  HvacRecircCtrl_Runnable_sys/4(HvacRecircRatPredAtStabyForCoolg_HvacRecircRatPredAtStabyForCoolg_write) -> HvacRecircRatPredAtStabyForCoolg_HvacRecircRatPredAtStabyForCoolg/1
  HvacRecircCtrl_Runnable_sys/5(HvacRecircRatReq_HvacRecircRatReq_write) -> HvacRecircRatReq_HvacRecircRatReq/1
  HvacRecircCtrl_Runnable/1 -> HvacRecircCtrl_Runnable_sys/Trigger

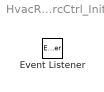
== system HvacRecircCtrl/HvacRecircCtrl_Init ==
  Event Listener : EventListener  EventName=reset

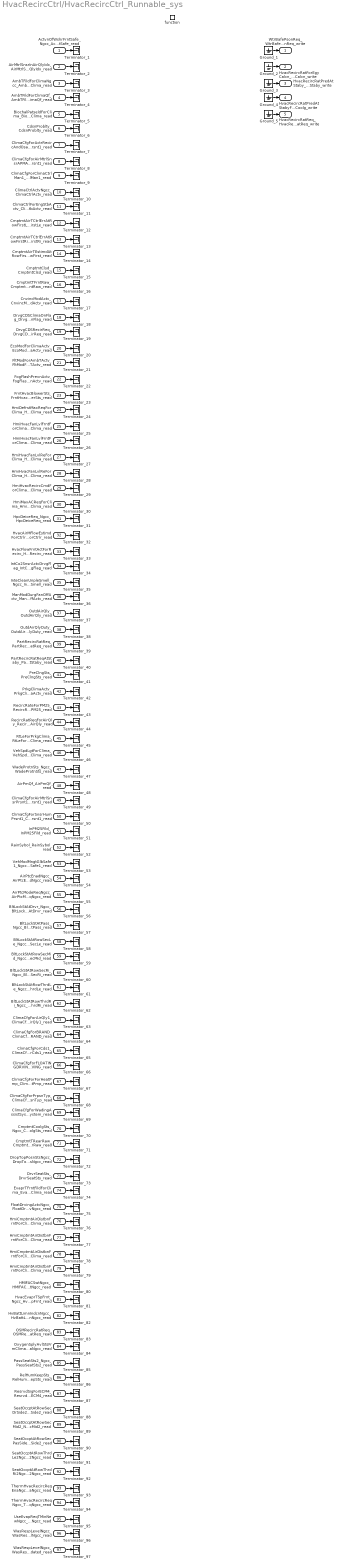
== system HvacRecircCtrl/HvacRecircCtrl_Runnable_sys ==
  ActvnOfWshrFrntSafe_Ngcc_ActvnOfWshrFrntSafe_read : Inport
  AirMtrlSnsrInAirQlyIdx_AirMtrlSnsrInAirQlyIdx_read : Inport  Port=2
  AmbTFildForClimaNgcc_AmbTFildForClima_read : Inport  Port=3
  AmbTFildForClimaQf_AmbTFildForClimaQf_read : Inport  Port=4
  BiochalPatseldForClima_BiochalPatseldForClima_read : Inport  Port=5
  CdsnProblty_CdsnProblty_read : Inport  Port=6
  ClimaCfgForActrRecircAndOsaCmnPrsnt1_ClimaCfgForActrRecircAndOsaCmnPrsnt1_read : Inport  Port=7
  ClimaCfgForAirMtrlSnsrAPMAPrsnt1_ClimaCfgForAirMtrlSnsrAPMAPrsnt1_read : Inport  Port=8
  ClimaCfgForClimaCtrlMan1_ClimaCfgForClimaCtrlMan1_read : Inport  Port=9
  ClimaCtrlActvNgcc_ClimaCtrlActv_read : Inport  Port=10
  ClimaCtrlForEngStbActv_ClimaCtrlForEngStbActv_read : Inport  Port=11
  CmptmtAirTCtrlErrAtRowFirstLe_CmptmtAirTCtrlErrAtRowFirstLe_read : Inport  Port=12
  CmptmtAirTCtrlErrAtRowFirstRi_CmptmtAirTCtrlErrAtRowFirstRi_read : Inport  Port=13
  CmptmtAirTEstimdAtRowFirst_CmptmtAirTEstimdAtRowFirst_read : Inport  Port=14
  CmptmtClsd_CmptmtClsd_read : Inport  Port=15
  CmptmtTFrntRaw_CmptmtTFrntRaw_read : Inport  Port=16
  CnvincModActv_CnvincModActv_read : Inport  Port=17
  DrvgCDSClimaOnFlag_DrvgCDSClimaOnFlag_read : Inport  Port=18
  DrvgCDSRecirReq_DrvgCDSRecirReq_read : Inport  Port=19
  EcoModForClimaActv_EcoModForClimaActv_read : Inport  Port=20
  FltModForAmbTActv_FltModForAmbTActv_read : Inport  Port=21
  FogFlashPrevnActv_FogFlashPrevnActv_read : Inport  Port=22
  FrntHvacBlowerSts_FrntHvacBlowerSts_read : Inport  Port=23
  HmiDefrstMaxReqForClima_HmiDefrstMaxReqForClima_read : Inport  Port=24
  HmiHvacFanLvlFrntForClima_HmiHvacFanLvlFrntForClima_read : Inport  Port=25
  HmiHvacFanLvlFrntForClima_HmiHvacFanLvlFrntRiForClima_read : Inport  Port=26
  HmiHvacFanLvlReForClima_HmiHvacFanLvlReForClima_read : Inport  Port=27
  HmiHvacFanLvlReForClima_HmiHvacFanLvlReRiForClima_read : Inport  Port=28
  HmiHvacRecircCmdForClima_HmiHvacRecircCmdForClima_read : Inport  Port=29
  HmiMaxACReqForClima_HmiMaxACReqForClima_read : Inport  Port=30
  HpcDeiceReq_Ngcc_HpcDeiceReq_read : Inport  Port=31
  HvacAirMFlowEstimdForCtrlr_HvacAirMFlowEstimdForCtrlr_read : Inport  Port=32
  HvacFlowFrntActForRecirc_HvacFlowFrntActForRecirc_read : Inport  Port=33
  IntCo2SnsrActvDrvgFlag_IntCo2SnsrActvDrvgFlag_read : Inport  Port=34
  InteCleanUnpleSmell_Ngcc_InteCleanUnpleSmell_read : Inport  Port=35
  ManModDurgFanOffActv_ManModDurgFanOffActv_read : Inport  Port=36
  OutdAirQly_OutdAirQly_read : Inport  Port=37
  OutdAirQlyDuty_OutdAirQlyDuty_read : Inport  Port=38
  PartRecircRatReq_PartRecircRatReq_read : Inport  Port=39
  PartRecircRatReqAtStaby_PartRecircRatReqAtStaby_read : Inport  Port=40
  PreClngSts_PreClngSts_read : Inport  Port=41
  PrkgClimaActv_PrkgClimaActv_read : Inport  Port=42
  RecircRateForPM25_RecircRateForPM25_read : Inport  Port=43
  RecircRatReqForAirQly_RecircRatReqForAirQly_read : Inport  Port=44
  RtLeForPrkgClima_RtLeForPrkgClima_read : Inport  Port=45
  VehSpdLgtForClima_VehSpdLgtForClima_read : Inport  Port=46
  WadeProtnSts_Ngcc_WadeProtnSts_read : Inport  Port=47
  AirPmQf_AirPmQf_read : Inport  Port=48
  ClimaCfgForAirMtrlSnsrPrsnt1_ClimaCfgForAirMtrlSnsrPrsnt1_read : Inport  Port=49
  ClimaCfgForSnsrHumPrsnt1_ClimaCfgForSnsrHumPrsnt1_read : Inport  Port=50
  InPM25Fild_InPM25Fild_read : Inport  Port=51
  RainSybol_RainSybol_read : Inport  Port=52
  VehModMngtGlbSafe1_Ngcc_VehModMngtGlbSafe1_read : Inport  Port=53
  AirPtcEnadNgcc_AirPtcEnadNgcc_read : Inport  Port=54
  AirPtcModeReqNgcc_AirPtcModeReqNgcc_read : Inport  Port=55
  BltLockStAtDrvr_Ngcc_BltLockStAtDrvr_read : Inport  Port=56
  BltLockStAtPass_Ngcc_BltLockStAtPass_read : Inport  Port=57
  BltLockStAtRowSecLe_Ngcc_BltLockStAtRowSecLe_read : Inport  Port=58
  BltLockStAtRowSecMid_Ngcc_BltLockStAtRowSecMid_read : Inport  Port=59
  BltLockStAtRowSecRi_Ngcc_BltLockStAtRowSecRi_read : Inport  Port=60
  BltLockStAtRowThrdLe_Ngcc_BltLockStAtRowThrdLe_read : Inport  Port=61
  BltLockStAtRowThrdRi_Ngcc_BltLockStAtRowThrdRi_read : Inport  Port=62
  ClimaCfgForAirQly1_ClimaCfgForAirQly1_read : Inport  Port=63
  ClimaCfgForBRAND_ClimaCfgForBRAND_read : Inport  Port=64
  ClimaCfgForCds1_ClimaCfgForCds1_read : Inport  Port=65
  ClimaCfgForFLOATINGDRVING_ClimaCfgForFLOATINGDRVING_read : Inport  Port=66
  ClimaCfgForForHeatPmp_ClimaCfgForForHeatPmp_read : Inport  Port=67
  ClimaCfgForPrpsnTyp_ClimaCfgForPrpsnTyp_read : Inport  Port=68
  ClimaCfgForWadingAssistSystem_ClimaCfgForWadingAssistSystem_read : Inport  Port=69
  CmptmtCoolgSts_Ngcc_CmptmtCoolgSts_read : Inport  Port=70
  CmptmtTRearRaw_CmptmtTRearRaw_read : Inport  Port=71
  DropTopPosnStsNgcc_DropTopPosnStsNgcc_read : Inport  Port=72
  DrvrSeatSts_DrvrSeatSts_read : Inport  Port=73
  EvaprTFrntFildForClima_EvaprTFrntFildForClima_read : Inport  Port=74
  FloatDrvingActvNgcc_FloatDrvingActvNgcc_read : Inport  Port=75
  HmiCmptmtAirDistbnFrntForClima_HmiCmptmtAirDistbnFrntForClima_read : Inport  Port=76
  HmiCmptmtAirDistbnFrntForClima_HmiCmptmtAirDistbnFrntRiForClima_read : Inport  Port=77
  HmiCmptmtAirDistbnFrntForClima_HmiCmptmtAirDistbnReForClima_read : Inport  Port=78
  HmiCmptmtAirDistbnFrntForClima_HmiCmptmtAirDistbnReRiForClima_read : Inport  Port=79
  HMIFACSwtNgcc_HMIFACSwtNgcc_read : Inport  Port=80
  HvacEvaprTSpFrnt_Ngcc_HvacEvaprTSpFrnt_read : Inport  Port=81
  HvBattLimnIndcnNgcc_HvBattLimnIndcnNgcc_read : Inport  Port=82
  OSMRecircRatReq_OSMRecircRatReq_read : Inport  Port=83
  OxygenSplyAvlStsFrmClimaNgcc_OxygenSplyAvlStsFrmClimaNgcc_read : Inport  Port=84
  PassSeatSts2_Ngcc_PassSeatSts2_read : Inport  Port=85
  RelHumKeepSts_RelHumKeepSts_read : Inport  Port=86
  ResrvdSigForECM4_ResrvdSigForECM4_read : Inport  Port=87
  SeatOccptAtRowSecDrSide2_Ngcc_SeatOccptAtRowSecDrSide2_read : Inport  Port=88
  SeatOccptAtRowSecMid2_Ngcc_SeatOccptAtRowSecMid2_read : Inport  Port=89
  SeatOccptAtRowSecPasSide2_Ngcc_SeatOccptAtRowSecPasSide2_read : Inport  Port=90
  SeatOccptAtRowThrdLe2Ngcc_SeatOccptAtRowThrdLe2Ngcc_read : Inport  Port=91
  SeatOccptAtRowThrdRi2Ngcc_SeatOccptAtRowThrdRi2Ngcc_read : Inport  Port=92
  ThermHvacRecircReqEnaNgcc_ThermHvacRecircReqEnaNgcc_read : Inport  Port=93
  ThermHvacRecircReqNgcc_ThermHvacRecircReqNgcc_read : Inport  Port=94
  UseEvapReqTMinNewNgcc_UseEvapReqTMinNewNgcc_read : Inport  Port=95
  WasRespLevelNgcc_WasRespLevelNgcc_read : Inport  Port=96
  WasRespLevelNgcc_WasRespLevelNgcc_IsUpdated_read : Inport  Port=97
  function : TriggerPort  TriggerType=function-call  VariantControl=(inherit)  SampleTimeType=periodic  SampleTime=-1
  Ground_{1..5} (5 alike) : Ground
  Terminator_{1..97} (97 alike) : Terminator
  WtrBafePsonReq_WtrBafePsonReq_write : Outport
      settings: EnsureOutportIsVirtual=on
  HvacRecircRatForEgyCalcn_HvacRecircRatForEgyCalcn_write : Outport  Port=2
      settings: EnsureOutportIsVirtual=on
  HvacRecircRatPredAtStaby_HvacRecircRatPredAtStaby_write : Outport  Port=3
      settings: EnsureOutportIsVirtual=on
  HvacRecircRatPredAtStabyForCoolg_HvacRecircRatPredAtStabyForCoolg_write : Outport  Port=4
      settings: EnsureOutportIsVirtual=on
  HvacRecircRatReq_HvacRecircRatReq_write : Outport  Port=5
      settings: EnsureOutportIsVirtual=on
  wires:
  ActvnOfWshrFrntSafe_Ngcc_ActvnOfWshrFrntSafe_read/1 -> Terminator_1/1
  AirMtrlSnsrInAirQlyIdx_AirMtrlSnsrInAirQlyIdx_read/1 -> Terminator_2/1
  AmbTFildForClimaNgcc_AmbTFildForClima_read/1 -> Terminator_3/1
  AmbTFildForClimaQf_AmbTFildForClimaQf_read/1 -> Terminator_4/1
  BiochalPatseldForClima_BiochalPatseldForClima_read/1 -> Terminator_5/1
  CdsnProblty_CdsnProblty_read/1 -> Terminator_6/1
  ClimaCfgForActrRecircAndOsaCmnPrsnt1_ClimaCfgForActrRecircAndOsaCmnPrsnt1_read/1 -> Terminator_7/1
  ClimaCfgForAirMtrlSnsrAPMAPrsnt1_ClimaCfgForAirMtrlSnsrAPMAPrsnt1_read/1 -> Terminator_8/1
  ClimaCfgForClimaCtrlMan1_ClimaCfgForClimaCtrlMan1_read/1 -> Terminator_9/1
  ClimaCtrlActvNgcc_ClimaCtrlActv_read/1 -> Terminator_10/1
  ClimaCtrlForEngStbActv_ClimaCtrlForEngStbActv_read/1 -> Terminator_11/1
  CmptmtAirTCtrlErrAtRowFirstLe_CmptmtAirTCtrlErrAtRowFirstLe_read/1 -> Terminator_12/1
  CmptmtAirTCtrlErrAtRowFirstRi_CmptmtAirTCtrlErrAtRowFirstRi_read/1 -> Terminator_13/1
  CmptmtAirTEstimdAtRowFirst_CmptmtAirTEstimdAtRowFirst_read/1 -> Terminator_14/1
  CmptmtClsd_CmptmtClsd_read/1 -> Terminator_15/1
  CmptmtTFrntRaw_CmptmtTFrntRaw_read/1 -> Terminator_16/1
  CnvincModActv_CnvincModActv_read/1 -> Terminator_17/1
  DrvgCDSClimaOnFlag_DrvgCDSClimaOnFlag_read/1 -> Terminator_18/1
  DrvgCDSRecirReq_DrvgCDSRecirReq_read/1 -> Terminator_19/1
  EcoModForClimaActv_EcoModForClimaActv_read/1 -> Terminator_20/1
  FltModForAmbTActv_FltModForAmbTActv_read/1 -> Terminator_21/1
  FogFlashPrevnActv_FogFlashPrevnActv_read/1 -> Terminator_22/1
  FrntHvacBlowerSts_FrntHvacBlowerSts_read/1 -> Terminator_23/1
  HmiDefrstMaxReqForClima_HmiDefrstMaxReqForClima_read/1 -> Terminator_24/1
  HmiHvacFanLvlFrntForClima_HmiHvacFanLvlFrntForClima_read/1 -> Terminator_25/1
  HmiHvacFanLvlFrntForClima_HmiHvacFanLvlFrntRiForClima_read/1 -> Terminator_26/1
  HmiHvacFanLvlReForClima_HmiHvacFanLvlReForClima_read/1 -> Terminator_27/1
  HmiHvacFanLvlReForClima_HmiHvacFanLvlReRiForClima_read/1 -> Terminator_28/1
  HmiHvacRecircCmdForClima_HmiHvacRecircCmdForClima_read/1 -> Terminator_29/1
  HmiMaxACReqForClima_HmiMaxACReqForClima_read/1 -> Terminator_30/1
  HpcDeiceReq_Ngcc_HpcDeiceReq_read/1 -> Terminator_31/1
  HvacAirMFlowEstimdForCtrlr_HvacAirMFlowEstimdForCtrlr_read/1 -> Terminator_32/1
  HvacFlowFrntActForRecirc_HvacFlowFrntActForRecirc_read/1 -> Terminator_33/1
  IntCo2SnsrActvDrvgFlag_IntCo2SnsrActvDrvgFlag_read/1 -> Terminator_34/1
  InteCleanUnpleSmell_Ngcc_InteCleanUnpleSmell_read/1 -> Terminator_35/1
  ManModDurgFanOffActv_ManModDurgFanOffActv_read/1 -> Terminator_36/1
  OutdAirQly_OutdAirQly_read/1 -> Terminator_37/1
  OutdAirQlyDuty_OutdAirQlyDuty_read/1 -> Terminator_38/1
  PartRecircRatReq_PartRecircRatReq_read/1 -> Terminator_39/1
  PartRecircRatReqAtStaby_PartRecircRatReqAtStaby_read/1 -> Terminator_40/1
  PreClngSts_PreClngSts_read/1 -> Terminator_41/1
  PrkgClimaActv_PrkgClimaActv_read/1 -> Terminator_42/1
  RecircRateForPM25_RecircRateForPM25_read/1 -> Terminator_43/1
  RecircRatReqForAirQly_RecircRatReqForAirQly_read/1 -> Terminator_44/1
  RtLeForPrkgClima_RtLeForPrkgClima_read/1 -> Terminator_45/1
  VehSpdLgtForClima_VehSpdLgtForClima_read/1 -> Terminator_46/1
  WadeProtnSts_Ngcc_WadeProtnSts_read/1 -> Terminator_47/1
  AirPmQf_AirPmQf_read/1 -> Terminator_48/1
  ClimaCfgForAirMtrlSnsrPrsnt1_ClimaCfgForAirMtrlSnsrPrsnt1_read/1 -> Terminator_49/1
  ClimaCfgForSnsrHumPrsnt1_ClimaCfgForSnsrHumPrsnt1_read/1 -> Terminator_50/1
  InPM25Fild_InPM25Fild_read/1 -> Terminator_51/1
  RainSybol_RainSybol_read/1 -> Terminator_52/1
  VehModMngtGlbSafe1_Ngcc_VehModMngtGlbSafe1_read/1 -> Terminator_53/1
  AirPtcEnadNgcc_AirPtcEnadNgcc_read/1 -> Terminator_54/1
  AirPtcModeReqNgcc_AirPtcModeReqNgcc_read/1 -> Terminator_55/1
  BltLockStAtDrvr_Ngcc_BltLockStAtDrvr_read/1 -> Terminator_56/1
  BltLockStAtPass_Ngcc_BltLockStAtPass_read/1 -> Terminator_57/1
  BltLockStAtRowSecLe_Ngcc_BltLockStAtRowSecLe_read/1 -> Terminator_58/1
  BltLockStAtRowSecMid_Ngcc_BltLockStAtRowSecMid_read/1 -> Terminator_59/1
  BltLockStAtRowSecRi_Ngcc_BltLockStAtRowSecRi_read/1 -> Terminator_60/1
  BltLockStAtRowThrdLe_Ngcc_BltLockStAtRowThrdLe_read/1 -> Terminator_61/1
  BltLockStAtRowThrdRi_Ngcc_BltLockStAtRowThrdRi_read/1 -> Terminator_62/1
  ClimaCfgForAirQly1_ClimaCfgForAirQly1_read/1 -> Terminator_63/1
  ClimaCfgForBRAND_ClimaCfgForBRAND_read/1 -> Terminator_64/1
  ClimaCfgForCds1_ClimaCfgForCds1_read/1 -> Terminator_65/1
  ClimaCfgForFLOATINGDRVING_ClimaCfgForFLOATINGDRVING_read/1 -> Terminator_66/1
  ClimaCfgForForHeatPmp_ClimaCfgForForHeatPmp_read/1 -> Terminator_67/1
  ClimaCfgForPrpsnTyp_ClimaCfgForPrpsnTyp_read/1 -> Terminator_68/1
  ClimaCfgForWadingAssistSystem_ClimaCfgForWadingAssistSystem_read/1 -> Terminator_69/1
  CmptmtCoolgSts_Ngcc_CmptmtCoolgSts_read/1 -> Terminator_70/1
  CmptmtTRearRaw_CmptmtTRearRaw_read/1 -> Terminator_71/1
  DropTopPosnStsNgcc_DropTopPosnStsNgcc_read/1 -> Terminator_72/1
  DrvrSeatSts_DrvrSeatSts_read/1 -> Terminator_73/1
  EvaprTFrntFildForClima_EvaprTFrntFildForClima_read/1 -> Terminator_74/1
  FloatDrvingActvNgcc_FloatDrvingActvNgcc_read/1 -> Terminator_75/1
  HmiCmptmtAirDistbnFrntForClima_HmiCmptmtAirDistbnFrntForClima_read/1 -> Terminator_76/1
  HmiCmptmtAirDistbnFrntForClima_HmiCmptmtAirDistbnFrntRiForClima_read/1 -> Terminator_77/1
  HmiCmptmtAirDistbnFrntForClima_HmiCmptmtAirDistbnReForClima_read/1 -> Terminator_78/1
  HmiCmptmtAirDistbnFrntForClima_HmiCmptmtAirDistbnReRiForClima_read/1 -> Terminator_79/1
  HMIFACSwtNgcc_HMIFACSwtNgcc_read/1 -> Terminator_80/1
  HvacEvaprTSpFrnt_Ngcc_HvacEvaprTSpFrnt_read/1 -> Terminator_81/1
  HvBattLimnIndcnNgcc_HvBattLimnIndcnNgcc_read/1 -> Terminator_82/1
  OSMRecircRatReq_OSMRecircRatReq_read/1 -> Terminator_83/1
  OxygenSplyAvlStsFrmClimaNgcc_OxygenSplyAvlStsFrmClimaNgcc_read/1 -> Terminator_84/1
  PassSeatSts2_Ngcc_PassSeatSts2_read/1 -> Terminator_85/1
  RelHumKeepSts_RelHumKeepSts_read/1 -> Terminator_86/1
  ResrvdSigForECM4_ResrvdSigForECM4_read/1 -> Terminator_87/1
  SeatOccptAtRowSecDrSide2_Ngcc_SeatOccptAtRowSecDrSide2_read/1 -> Terminator_88/1
  SeatOccptAtRowSecMid2_Ngcc_SeatOccptAtRowSecMid2_read/1 -> Terminator_89/1
  SeatOccptAtRowSecPasSide2_Ngcc_SeatOccptAtRowSecPasSide2_read/1 -> Terminator_90/1
  SeatOccptAtRowThrdLe2Ngcc_SeatOccptAtRowThrdLe2Ngcc_read/1 -> Terminator_91/1
  SeatOccptAtRowThrdRi2Ngcc_SeatOccptAtRowThrdRi2Ngcc_read/1 -> Terminator_92/1
  ThermHvacRecircReqEnaNgcc_ThermHvacRecircReqEnaNgcc_read/1 -> Terminator_93/1
  ThermHvacRecircReqNgcc_ThermHvacRecircReqNgcc_read/1 -> Terminator_94/1
  UseEvapReqTMinNewNgcc_UseEvapReqTMinNewNgcc_read/1 -> Terminator_95/1
  WasRespLevelNgcc_WasRespLevelNgcc_read/1 -> Terminator_96/1
  WasRespLevelNgcc_WasRespLevelNgcc_IsUpdated_read/1 -> Terminator_97/1
  Ground_1/1 -> WtrBafePsonReq_WtrBafePsonReq_write/1
  Ground_2/1 -> HvacRecircRatForEgyCalcn_HvacRecircRatForEgyCalcn_write/1
  Ground_3/1 -> HvacRecircRatPredAtStaby_HvacRecircRatPredAtStaby_write/1
  Ground_4/1 -> HvacRecircRatPredAtStabyForCoolg_HvacRecircRatPredAtStabyForCoolg_write/1
  Ground_5/1 -> HvacRecircRatReq_HvacRecircRatReq_write/1
